simulink model "MX_Gateway"  (Simulink 9.0)
settings: Solver=FixedStepDiscrete  StartTime=0.0  StopTime=100  FixedStep=0.01  RelTol=1e-3
values from neighbouring files (symbols this model uses):
  ACTIVE = Bobcat.Parameter; CreateDefine( ACTIVE                         ,1                          ,'Define' ,'defines.h' )    [defines.m]
  ACTIVE_MODE_ONE = Bobcat.Parameter; CreateDefine( ACTIVE_MODE_ONE                ,19                         ,'Define' ,'defines.h' )    [defines.m]
  AUTHENTICATED = Bobcat.Parameter; CreateDefine( AUTHENTICATED                  ,1                          ,'Define' ,'defines.h' )    [defines.m]
  AUX_EMEA_CONTROLS = Bobcat.Parameter; CreateDefine( AUX_EMEA_CONTROLS  ,1   ,'Define' ,'defines.h' )    [enums.m]
  AUX_FLOW_HIGH = Bobcat.Parameter; CreateDefine( AUX_FLOW_HIGH           ,4  ,'Define' ,'defines.h' )    [enums.m]
  AUX_FLOW_INACTIVE = Bobcat.Parameter; CreateDefine( AUX_FLOW_INACTIVE       ,0  ,'Define' ,'defines.h' )    [enums.m]
  AUX_FLOW_LOW = Bobcat.Parameter; CreateDefine( AUX_FLOW_LOW            ,1  ,'Define' ,'defines.h' )    [enums.m]
  AUX_FLOW_MEDIUM = Bobcat.Parameter; CreateDefine( AUX_FLOW_MEDIUM         ,2  ,'Define' ,'defines.h' )    [enums.m]
  AUX_FLOW_STANDARD = Bobcat.Parameter; CreateDefine( AUX_FLOW_STANDARD       ,3  ,'Define' ,'defines.h' )    [enums.m]
  AUX_NON_FUNCTIONAL = Bobcat.Parameter; CreateDefine( AUX_NON_FUNCTIONAL ,255 ,'Define' ,'defines.h' )    [enums.m]
  BASE_ACTIVE = Bobcat.Parameter; CreateDefine( BASE_ACTIVE                    ,253                        ,'Define' ,'defines.h' )    [defines.m]
  BASE_DETENT = Bobcat.Parameter; CreateDefine( BASE_DETENT                    ,1                          ,'Define' ,'defines.h' )    [defines.m]
  BEEP_NONE = Bobcat.Parameter; CreateDefine( BEEP_NONE                      ,255                        ,'Define' ,'defines.h' )    [defines.m]
  CD_AIR_FILTER_DEBOUNCE_NORMAL = Bobcat.Parameter; CreateAliasCD( CD_AIR_FILTER_DEBOUNCE_NORMAL                        ,uint16(500)                ,'ConstantDataPointer' ,'CONSTANT_DATA' ,'AIR_FILTER_DEBOUNCE_NORMAL')    [cdata.m]    (also in hc12_cdata.m; m4_cdata.m)
  CD_AIR_FILTER_DEBOUNCE_NOT_CONNECTED = Bobcat.Parameter; CreateAliasCD( CD_AIR_FILTER_DEBOUNCE_NOT_CONNECTED                 ,uint16(3000)               ,'ConstantDataPointer' ,'CONSTANT_DATA' ,'AIR_FILTER_DEBOUNCE_NOT_CONNECTED')    [cdata.m]    (also in hc12_cdata.m; m4_cdata.m)
  CD_AIR_FILTER_DEBOUNCE_PLUGGED = Bobcat.Parameter; CreateAliasCD( CD_AIR_FILTER_DEBOUNCE_PLUGGED                       ,uint16(3000)               ,'ConstantDataPointer' ,'CONSTANT_DATA' ,'AIR_FILTER_DEBOUNCE_PLUGGED')    [cdata.m]    (also in hc12_cdata.m; m4_cdata.m)
  CD_AIR_FILTER_FUNCTION = Bobcat.Parameter; CreateAliasCD( CD_AIR_FILTER_FUNCTION                               ,uint8(255)                 ,'ConstantDataPointer' ,'CONSTANT_DATA' ,'AIR_FILTER_FUNCTION')    [cdata.m]    (also in hc12_cdata.m; m4_cdata.m)
  CD_AIR_FILTER_PLUGGED_WEIGHT = Bobcat.Parameter; CreateAliasCD( CD_AIR_FILTER_PLUGGED_WEIGHT                         ,uint8(2)                   ,'ConstantDataPointer' ,'CONSTANT_DATA' ,'AIR_FILTER_PLUGGED_WEIGHT')    [cdata.m]    (also in hc12_cdata.m; m4_cdata.m)
  CD_AIR_FILTER_UNPLUGGED_WEIGHT = Bobcat.Parameter; CreateAliasCD( CD_AIR_FILTER_UNPLUGGED_WEIGHT                       ,uint8(1)                   ,'ConstantDataPointer' ,'CONSTANT_DATA' ,'AIR_FILTER_UNPLUGGED_WEIGHT')    [cdata.m]    (also in hc12_cdata.m; m4_cdata.m)
  CD_AUTOIDLE_ARMBAR_DELAY = Bobcat.Parameter; CreateAliasCD( CD_AUTOIDLE_ARMBAR_DELAY                             ,uint8(2)                   ,'ConstantDataPointer' ,'CONSTANT_DATA' ,'AUTOIDLE_ARMBAR_DELAY')    [cdata.m]    (also in hc12_cdata.m; m4_cdata.m)
  CD_AUTOIDLE_FUNCTION = Bobcat.Parameter; CreateAliasCD( CD_AUTOIDLE_FUNCTION                                 ,uint8(255)                 ,'ConstantDataPointer' ,'CONSTANT_DATA' ,'AUTOIDLE_FUNCTION')    [cdata.m]    (also in hc12_cdata.m; m4_cdata.m)
  CD_AUTOIDLE_IDLE_DELAY = Bobcat.Parameter; CreateAliasCD( CD_AUTOIDLE_IDLE_DELAY                               ,uint8(40)                  ,'ConstantDataPointer' ,'CONSTANT_DATA' ,'AUTOIDLE_IDLE_DELAY')    [cdata.m]    (also in hc12_cdata.m; m4_cdata.m)
  CD_AUTOIDLE_LOW_IDLE_START = Bobcat.Parameter; CreateAliasCD( CD_AUTOIDLE_LOW_IDLE_START                           ,uint8(255)                 ,'ConstantDataPointer' ,'CONSTANT_DATA' ,'AUTOIDLE_LOW_IDLE_START')    [cdata.m]    (also in hc12_cdata.m; m4_cdata.m)
  CD_AUTOIDLE_PRESSURE_OFF = Bobcat.Parameter; CreateAliasCD( CD_AUTOIDLE_PRESSURE_OFF                             ,uint16(300)                ,'ConstantDataPointer' ,'CONSTANT_DATA' ,'AUTOIDLE_PRESSURE_OFF')    [cdata.m]    (also in hc12_cdata.m; m4_cdata.m)
  CD_AUTOIDLE_PRESSURE_ON = Bobcat.Parameter; CreateAliasCD( CD_AUTOIDLE_PRESSURE_ON                              ,uint16(270)                ,'ConstantDataPointer' ,'CONSTANT_DATA' ,'AUTOIDLE_PRESSURE_ON')    [cdata.m]    (also in hc12_cdata.m; m4_cdata.m)
  CD_AUTOSHIFT_FUNCTION = Bobcat.Parameter; CreateAliasCD( CD_AUTOSHIFT_FUNCTION                                ,uint8(255)                 ,'ConstantDataPointer' ,'CONSTANT_DATA' ,'AUTOSHIFT_FUNCTION')    [cdata.m]    (also in hc12_cdata.m; m4_cdata.m)
  CD_AUTOSHIFT_PHIGH1 = Bobcat.Parameter; CreateAliasCD( CD_AUTOSHIFT_PHIGH1                                  ,uint16(205)                ,'ConstantDataPointer' ,'CONSTANT_DATA' ,'AUTOSHIFT_PHIGH1')    [cdata.m]    (also in hc12_cdata.m; m4_cdata.m)
  CD_AUTOSHIFT_PHIGH2 = Bobcat.Parameter; CreateAliasCD( CD_AUTOSHIFT_PHIGH2                                  ,uint16(193)                ,'ConstantDataPointer' ,'CONSTANT_DATA' ,'AUTOSHIFT_PHIGH2')    [cdata.m]    (also in hc12_cdata.m; m4_cdata.m)
  CD_AUTOSHIFT_PHIGH_TEMP1 = Bobcat.Parameter; CreateAliasCD( CD_AUTOSHIFT_PHIGH_TEMP1                             ,int16(70)                  ,'ConstantDataPointer' ,'CONSTANT_DATA' ,'AUTOSHIFT_PHIGH_TEMP1')    [cdata.m]    (also in hc12_cdata.m; m4_cdata.m)
  CD_AUTOSHIFT_PHIGH_TEMP2 = Bobcat.Parameter; CreateAliasCD( CD_AUTOSHIFT_PHIGH_TEMP2                             ,int16(160)                 ,'ConstantDataPointer' ,'CONSTANT_DATA' ,'AUTOSHIFT_PHIGH_TEMP2')    [cdata.m]    (also in hc12_cdata.m; m4_cdata.m)
  CD_AUTOSHIFT_PLOW1 = Bobcat.Parameter; CreateAliasCD( CD_AUTOSHIFT_PLOW1                                   ,uint16(130)                ,'ConstantDataPointer' ,'CONSTANT_DATA' ,'AUTOSHIFT_PLOW1')    [cdata.m]    (also in hc12_cdata.m; m4_cdata.m)
  CD_AUTOSHIFT_PLOW2 = Bobcat.Parameter; CreateAliasCD( CD_AUTOSHIFT_PLOW2                                   ,uint16(100)                ,'ConstantDataPointer' ,'CONSTANT_DATA' ,'AUTOSHIFT_PLOW2')    [cdata.m]    (also in hc12_cdata.m; m4_cdata.m)
  CD_AUTOSHIFT_PLOW_TEMP1 = Bobcat.Parameter; CreateAliasCD( CD_AUTOSHIFT_PLOW_TEMP1                              ,int16(20)                  ,'ConstantDataPointer' ,'CONSTANT_DATA' ,'AUTOSHIFT_PLOW_TEMP1')    [cdata.m]    (also in hc12_cdata.m; m4_cdata.m)
  CD_AUTOSHIFT_PLOW_TEMP2 = Bobcat.Parameter; CreateAliasCD( CD_AUTOSHIFT_PLOW_TEMP2                              ,int16(80)                  ,'ConstantDataPointer' ,'CONSTANT_DATA' ,'AUTOSHIFT_PLOW_TEMP2')    [cdata.m]    (also in hc12_cdata.m; m4_cdata.m)
  CD_AUTOSHIFT_TRAVEL_DELAY = Bobcat.Parameter; CreateAliasCD( CD_AUTOSHIFT_TRAVEL_DELAY                            ,uint8(32)                  ,'ConstantDataPointer' ,'CONSTANT_DATA' ,'AUTOSHIFT_TRAVEL_DELAY')    [cdata.m]    (also in hc12_cdata.m; m4_cdata.m)
  CD_AUX2_CRACKPOINT_CURRENT = Bobcat.Parameter; CreateAliasCD( CD_AUX2_CRACKPOINT_CURRENT                           ,fi(0,0,16,12,'hex','0c28') ,'ConstantDataPointer' ,'CONSTANT_DATA' ,'AUX2_CRACKPOINT_CURRENT')    [cdata.m]    (also in hc12_cdata.m; m4_cdata.m)
  CD_AUX2_FLOW_LOW_FUNCTION = Bobcat.Parameter; CreateAliasCD( CD_AUX2_FLOW_LOW_FUNCTION                            ,uint8(255)                 ,'ConstantDataPointer' ,'CONSTANT_DATA' ,'AUX2_FLOW_LOW_FUNCTION')    [cdata.m]    (also in hc12_cdata.m; m4_cdata.m)
  CD_AUX2_FLOW_MED_FUNCTION = Bobcat.Parameter; CreateAliasCD( CD_AUX2_FLOW_MED_FUNCTION                            ,uint8(255)                 ,'ConstantDataPointer' ,'CONSTANT_DATA' ,'AUX2_FLOW_MED_FUNCTION')    [cdata.m]    (also in hc12_cdata.m; m4_cdata.m)
  CD_AUX2_HI_FLOW_PERCENT = Bobcat.Parameter; CreateAliasCD( CD_AUX2_HI_FLOW_PERCENT                              ,fi(0,0,16,16,'hex','ffff') ,'ConstantDataPointer' ,'CONSTANT_DATA' ,'AUX2_HI_FLOW_PERCENT')    [cdata.m]    (also in hc12_cdata.m; m4_cdata.m)
  CD_AUX2_LOW_FLOW_PERCENT = Bobcat.Parameter; CreateAliasCD( CD_AUX2_LOW_FLOW_PERCENT                             ,fi(0,0,16,16,'hex','7333') ,'ConstantDataPointer' ,'CONSTANT_DATA' ,'AUX2_LOW_FLOW_PERCENT')    [cdata.m]    (also in hc12_cdata.m; m4_cdata.m)
  CD_AUX2_MAX_FLOW_CURRENT = Bobcat.Parameter; CreateAliasCD( CD_AUX2_MAX_FLOW_CURRENT                             ,fi(0,0,16,12,'hex','14cc') ,'ConstantDataPointer' ,'CONSTANT_DATA' ,'AUX2_MAX_FLOW_CURRENT')    [cdata.m]    (also in hc12_cdata.m; m4_cdata.m)
  CD_AUX2_MED_FLOW_PERCENT = Bobcat.Parameter; CreateAliasCD( CD_AUX2_MED_FLOW_PERCENT                             ,fi(0,0,16,16,'hex','9999') ,'ConstantDataPointer' ,'CONSTANT_DATA' ,'AUX2_MED_FLOW_PERCENT')    [cdata.m]    (also in hc12_cdata.m; m4_cdata.m)
  CD_AUX_CRACKPOINT_CURRENT = Bobcat.Parameter; CreateAliasCD( CD_AUX_CRACKPOINT_CURRENT                            ,fi(0,0,16,12,'hex','0000') ,'ConstantDataPointer' ,'CONSTANT_DATA' ,'AUX_CRACKPOINT_CURRENT')    [cdata.m]    (also in hc12_cdata.m; m4_cdata.m)
  CD_AUX_DETENT_FLASH_TIME = Bobcat.Parameter; CreateAliasCD( CD_AUX_DETENT_FLASH_TIME                             ,uint16(72)                 ,'ConstantDataPointer' ,'CONSTANT_DATA' ,'AUX_DETENT_FLASH_TIME')    [cdata.m]    (also in hc12_cdata.m; m4_cdata.m)
  CD_AUX_DETENT_HOLD_TIME = Bobcat.Parameter; CreateAliasCD( CD_AUX_DETENT_HOLD_TIME                              ,uint16(100)                ,'ConstantDataPointer' ,'CONSTANT_DATA' ,'AUX_DETENT_HOLD_TIME')    [cdata.m]    (also in hc12_cdata.m; m4_cdata.m)
  CD_AUX_FULL_ON_AUX1 = Bobcat.Parameter; CreateAliasCD( CD_AUX_FULL_ON_AUX1                                  ,fi(0,0,16,12,'hex','10cd') ,'ConstantDataPointer' ,'CONSTANT_DATA' ,'AUX_FULL_ON_AUX1')    [cdata.m]    (also in hc12_cdata.m; m4_cdata.m)
  CD_AUX_FULL_ON_AUX2 = Bobcat.Parameter; CreateAliasCD( CD_AUX_FULL_ON_AUX2                                  ,fi(0,0,16,12,'hex','139d') ,'ConstantDataPointer' ,'CONSTANT_DATA' ,'AUX_FULL_ON_AUX2')    [cdata.m]    (also in hc12_cdata.m; m4_cdata.m)
  CD_AUX_FULL_ON_AUX3 = Bobcat.Parameter; CreateAliasCD( CD_AUX_FULL_ON_AUX3                                  ,fi(0,0,16,12,'hex','17d7') ,'ConstantDataPointer' ,'CONSTANT_DATA' ,'AUX_FULL_ON_AUX3')    [cdata.m]    (also in hc12_cdata.m; m4_cdata.m)
  CD_AUX_FUNCTION = Bobcat.Parameter; CreateAliasCD( CD_AUX_FUNCTION                                      ,uint8(0)                   ,'ConstantDataPointer' ,'CONSTANT_DATA' ,'AUX_FUNCTION')    [cdata.m]    (also in hc12_cdata.m; m4_cdata.m)
  CD_AUX_RATE_LIMIT_CRACKPOINT = Bobcat.Parameter; CreateAliasCD( CD_AUX_RATE_LIMIT_CRACKPOINT                         ,fi(0,0,16,12,'hex','0800') ,'ConstantDataPointer' ,'CONSTANT_DATA' ,'AUX_RATE_LIMIT_CRACKPOINT')    [cdata.m]    (also in hc12_cdata.m; m4_cdata.m)
  CD_AUX_RATE_LIMIT_CRACKPOINT_RATE = Bobcat.Parameter; CreateAliasCD( CD_AUX_RATE_LIMIT_CRACKPOINT_RATE                    ,fi(0,1,32,12,'int',102400) ,'ConstantDataPointer' ,'CONSTANT_DATA' ,'AUX_RATE_LIMIT_CRACKPOINT_RATE')    [cdata.m]    (also in hc12_cdata.m; m4_cdata.m)
  CD_AUX_RATE_LIMIT_FUNCTION = Bobcat.Parameter; CreateAliasCD( CD_AUX_RATE_LIMIT_FUNCTION                           ,uint8(255)                 ,'ConstantDataPointer' ,'CONSTANT_DATA' ,'AUX_RATE_LIMIT_FUNCTION')    [cdata.m]    (also in hc12_cdata.m; m4_cdata.m)
  CD_AUX_RATE_LIMIT_MAX_ENG_SPD = Bobcat.Parameter; CreateAliasCD( CD_AUX_RATE_LIMIT_MAX_ENG_SPD                        ,uint16(1600)               ,'ConstantDataPointer' ,'CONSTANT_DATA' ,'AUX_RATE_LIMIT_MAX_ENG_SPD')    [cdata.m]    (also in hc12_cdata.m; m4_cdata.m)
  CD_AUX_RATE_LIMIT_MIN_ENG_SPD = Bobcat.Parameter; CreateAliasCD( CD_AUX_RATE_LIMIT_MIN_ENG_SPD                        ,uint16(100)                ,'ConstantDataPointer' ,'CONSTANT_DATA' ,'AUX_RATE_LIMIT_MIN_ENG_SPD')    [cdata.m]    (also in hc12_cdata.m; m4_cdata.m)
  CD_AUX_RATE_LIMIT_NORMAL_RATE = Bobcat.Parameter; CreateAliasCD( CD_AUX_RATE_LIMIT_NORMAL_RATE                        ,fi(0,1,32,12,'int',2048)   ,'ConstantDataPointer' ,'CONSTANT_DATA' ,'AUX_RATE_LIMIT_NORMAL_RATE')    [cdata.m]    (also in hc12_cdata.m; m4_cdata.m)
  CD_AUXPOT_CAL_DEADBAND_HIGH = Bobcat.Parameter; CreateAliasCD( CD_AUXPOT_CAL_DEADBAND_HIGH                          ,uint16(574)                ,'ConstantDataPointer' ,'CONSTANT_DATA' ,'AUXPOT_CAL_DEADBAND_HIGH')    [cdata.m]    (also in hc12_cdata.m; m4_cdata.m)
  CD_AUXPOT_CAL_DEADBAND_LOW = Bobcat.Parameter; CreateAliasCD( CD_AUXPOT_CAL_DEADBAND_LOW                           ,uint16(424)                ,'ConstantDataPointer' ,'CONSTANT_DATA' ,'AUXPOT_CAL_DEADBAND_LOW')    [cdata.m]    (also in hc12_cdata.m; m4_cdata.m)
  CD_AUXPOT_CENTER_RATIO = Bobcat.Parameter; CreateAliasCD( CD_AUXPOT_CENTER_RATIO                               ,uint16(500)                ,'ConstantDataPointer' ,'CONSTANT_DATA' ,'AUXPOT_CENTER_RATIO')    [cdata.m]    (also in hc12_cdata.m; m4_cdata.m)
  CD_AUXPOT_DEADBAND = Bobcat.Parameter; CreateAliasCD( CD_AUXPOT_DEADBAND                                   ,uint8(38)                  ,'ConstantDataPointer' ,'CONSTANT_DATA' ,'AUXPOT_DEADBAND')    [cdata.m]    (also in hc12_cdata.m; m4_cdata.m)
  CD_AUXPOT_FUNCTION = Bobcat.Parameter; CreateAliasCD( CD_AUXPOT_FUNCTION                                   ,uint8(0)                   ,'ConstantDataPointer' ,'CONSTANT_DATA' ,'AUXPOT_FUNCTION')    [cdata.m]    (also in hc12_cdata.m; m4_cdata.m)
  CD_AUXPOT_RATIO_GAIN = Bobcat.Parameter; CreateAliasCD( CD_AUXPOT_RATIO_GAIN                                 ,uint16(1000)               ,'ConstantDataPointer' ,'CONSTANT_DATA' ,'AUXPOT_RATIO_GAIN')    [cdata.m]    (also in hc12_cdata.m; m4_cdata.m)
  CD_CAN_THROTTLE_FUNCTION = Bobcat.Parameter; CreateAliasCD( CD_CAN_THROTTLE_FUNCTION                             ,uint8(255)                 ,'ConstantDataPointer' ,'CONSTANT_DATA' ,'CAN_THROTTLE_FUNCTION')    [cdata.m]    (also in hc12_cdata.m; m4_cdata.m)
  CD_CAN_THROTTLE_REVERSE = Bobcat.Parameter; CreateAliasCD( CD_CAN_THROTTLE_REVERSE                              ,uint8(255)                 ,'ConstantDataPointer' ,'CONSTANT_DATA' ,'CAN_THROTTLE_REVERSE')    [cdata.m]    (also in hc12_cdata.m; m4_cdata.m)
  CD_CONSOLE_DEBOUNCE_ABSENT = Bobcat.Parameter; CreateAliasCD( CD_CONSOLE_DEBOUNCE_ABSENT                           ,uint16(12)                 ,'ConstantDataPointer' ,'CONSTANT_DATA' ,'CONSOLE_DEBOUNCE_ABSENT')    [cdata.m]    (also in hc12_cdata.m; m4_cdata.m)
  CD_CONSOLE_DEBOUNCE_OORH = Bobcat.Parameter; CreateAliasCD( CD_CONSOLE_DEBOUNCE_OORH                             ,uint16(12)                 ,'ConstantDataPointer' ,'CONSTANT_DATA' ,'CONSOLE_DEBOUNCE_OORH')    [cdata.m]    (also in hc12_cdata.m; m4_cdata.m)
  CD_CONSOLE_DEBOUNCE_OORL = Bobcat.Parameter; CreateAliasCD( CD_CONSOLE_DEBOUNCE_OORL                             ,uint16(12)                 ,'ConstantDataPointer' ,'CONSTANT_DATA' ,'CONSOLE_DEBOUNCE_OORL')    [cdata.m]    (also in hc12_cdata.m; m4_cdata.m)
  CD_CONSOLE_DEBOUNCE_PRESENT = Bobcat.Parameter; CreateAliasCD( CD_CONSOLE_DEBOUNCE_PRESENT                          ,uint16(12)                 ,'ConstantDataPointer' ,'CONSTANT_DATA' ,'CONSOLE_DEBOUNCE_PRESENT')    [cdata.m]    (also in hc12_cdata.m; m4_cdata.m)
  CD_CONSOLE_FUNCTION = Bobcat.Parameter; CreateAliasCD( CD_CONSOLE_FUNCTION                                  ,uint8(0)                   ,'ConstantDataPointer' ,'CONSTANT_DATA' ,'CONSOLE_FUNCTION')    [cdata.m]    (also in hc12_cdata.m; m4_cdata.m)
  CD_CONSOLE_OORH = Bobcat.Parameter; CreateAliasCD( CD_CONSOLE_OORH                                      ,uint16(740)                ,'ConstantDataPointer' ,'CONSTANT_DATA' ,'CONSOLE_OORH')    [cdata.m]    (also in hc12_cdata.m; m4_cdata.m)
  CD_CONSOLE_OORL = Bobcat.Parameter; CreateAliasCD( CD_CONSOLE_OORL                                      ,uint16(10)                 ,'ConstantDataPointer' ,'CONSTANT_DATA' ,'CONSOLE_OORL')    [cdata.m]    (also in hc12_cdata.m; m4_cdata.m)
  CD_CONSOLE_PRESENT_HIGH = Bobcat.Parameter; CreateAliasCD( CD_CONSOLE_PRESENT_HIGH                              ,uint16(515)                ,'ConstantDataPointer' ,'CONSTANT_DATA' ,'CONSOLE_PRESENT_HIGH')    [cdata.m]    (also in hc12_cdata.m; m4_cdata.m)
  CD_CONSOLE_PRESENT_LOW = Bobcat.Parameter; CreateAliasCD( CD_CONSOLE_PRESENT_LOW                               ,uint16(375)                ,'ConstantDataPointer' ,'CONSTANT_DATA' ,'CONSOLE_PRESENT_LOW')    [cdata.m]    (also in hc12_cdata.m; m4_cdata.m)
  CD_DELUXE_GEN5_5_FUNCTION = Bobcat.Parameter; CreateAliasCD( CD_DELUXE_GEN5_5_FUNCTION                            ,uint8(255)                 ,'ConstantDataPointer' ,'CONSTANT_DATA' ,'DELUXE_GEN5_5_FUNCTION')    [cdata.m]    (also in hc12_cdata.m; m4_cdata.m)
  CD_DELUXE_GEN5_FUNCTION = Bobcat.Parameter; CreateAliasCD( CD_DELUXE_GEN5_FUNCTION                              ,uint8(255)                 ,'ConstantDataPointer' ,'CONSTANT_DATA' ,'DELUXE_GEN5_FUNCTION')    [cdata.m]    (also in hc12_cdata.m; m4_cdata.m)
  CD_DERATE_ENGINE_SPEED_DEBOUNCE_HIGH = Bobcat.Parameter; CreateAliasCD( CD_DERATE_ENGINE_SPEED_DEBOUNCE_HIGH                 ,uint16(200)                ,'ConstantDataPointer' ,'CONSTANT_DATA' ,'DERATE_ENGINE_SPEED_DEBOUNCE_HIGH')    [cdata.m]    (also in hc12_cdata.m; m4_cdata.m)
  CD_DERATE_ENGINE_SPEED_DEBOUNCE_SHUTDOWN = Bobcat.Parameter; CreateAliasCD( CD_DERATE_ENGINE_SPEED_DEBOUNCE_SHUTDOWN             ,uint16(1000)               ,'ConstantDataPointer' ,'CONSTANT_DATA' ,'DERATE_ENGINE_SPEED_DEBOUNCE_SHUTDOWN')    [cdata.m]    (also in hc12_cdata.m; m4_cdata.m)
  CD_DERATE_ENGINE_SPEED_HIGH_OFFSET = Bobcat.Parameter; CreateAliasCD( CD_DERATE_ENGINE_SPEED_HIGH_OFFSET                   ,uint16(200)                ,'ConstantDataPointer' ,'CONSTANT_DATA' ,'DERATE_ENGINE_SPEED_HIGH_OFFSET')    [cdata.m]    (also in hc12_cdata.m; m4_cdata.m)
  CD_DERATE_METHOD = Bobcat.Parameter; CreateAliasCD( CD_DERATE_METHOD                                     ,uint8(0)                   ,'ConstantDataPointer' ,'CONSTANT_DATA' ,'DERATE_METHOD')    [cdata.m]    (also in hc12_cdata.m; m4_cdata.m)
  CD_DUMP_VALVE_FUNCTION = Bobcat.Parameter; CreateAliasCD( CD_DUMP_VALVE_FUNCTION                               ,uint8(255)                 ,'ConstantDataPointer' ,'CONSTANT_DATA' ,'DUMP_VALVE_FUNCTION')    [cdata.m]    (also in hc12_cdata.m; m4_cdata.m)
  CD_DUMP_VALVE_ON_CURRENT = Bobcat.Parameter; CreateAliasCD( CD_DUMP_VALVE_ON_CURRENT                             ,fi(0,0,16,12,'hex','ffff') ,'ConstantDataPointer' ,'CONSTANT_DATA' ,'DUMP_VALVE_ON_CURRENT')    [cdata.m]    (also in hc12_cdata.m; m4_cdata.m)
  CD_ECO_MODE_THROTTLE_REDUCTION = Bobcat.Parameter; CreateAliasCD( CD_ECO_MODE_THROTTLE_REDUCTION                       ,uint16(850)                ,'ConstantDataPointer' ,'CONSTANT_DATA' ,'ECO_MODE_THROTTLE_REDUCTION')    [cdata.m]    (also in hc12_cdata.m; m4_cdata.m)
  CD_ECT_FILTER_GAIN = Bobcat.Parameter; CreateAliasCD( CD_ECT_FILTER_GAIN                                   ,uint8(6)                   ,'ConstantDataPointer' ,'CONSTANT_DATA' ,'ECT_FILTER_GAIN')    [cdata.m]    (also in hc12_cdata.m; m4_cdata.m)
  CD_ECU_FUNCTION = Bobcat.Parameter; CreateAliasCD( CD_ECU_FUNCTION                                      ,uint8(255)                 ,'ConstantDataPointer' ,'CONSTANT_DATA' ,'ECU_FUNCTION')    [cdata.m]    (also in hc12_cdata.m; m4_cdata.m)
  CD_ECU_HOLD_DEBOUNCE_ERROR_ON = Bobcat.Parameter; CreateAliasCD( CD_ECU_HOLD_DEBOUNCE_ERROR_ON                        ,uint16(20)                 ,'ConstantDataPointer' ,'CONSTANT_DATA' ,'ECU_HOLD_DEBOUNCE_ERROR_ON')    [cdata.m]    (also in hc12_cdata.m; m4_cdata.m)
  CD_EMEA_CONTROLS_HOLD_TIME = Bobcat.Parameter; CreateAliasCD( CD_EMEA_CONTROLS_HOLD_TIME                           ,uint16(30)                 ,'ConstantDataPointer' ,'CONSTANT_DATA' ,'EMEA_CONTROLS_HOLD_TIME')    [cdata.m]    (also in hc12_cdata.m; m4_cdata.m)
  CD_EMEA_CONTROLS_OPTION_A = Bobcat.Parameter; CreateAliasCD( CD_EMEA_CONTROLS_OPTION_A                            ,uint8(255)                 ,'ConstantDataPointer' ,'CONSTANT_DATA' ,'EMEA_CONTROLS_OPTION_A')    [cdata.m]    (also in hc12_cdata.m; m4_cdata.m)
  CD_EMEA_CONTROLS_OPTION_C = Bobcat.Parameter; CreateAliasCD( CD_EMEA_CONTROLS_OPTION_C                            ,uint8(255)                 ,'ConstantDataPointer' ,'CONSTANT_DATA' ,'EMEA_CONTROLS_OPTION_C')    [cdata.m]    (also in hc12_cdata.m; m4_cdata.m)
  CD_EMEA_CONTROLS_REVERSE = Bobcat.Parameter; CreateAliasCD( CD_EMEA_CONTROLS_REVERSE                             ,uint8(255)                 ,'ConstantDataPointer' ,'CONSTANT_DATA' ,'EMEA_CONTROLS_REVERSE')    [cdata.m]    (also in hc12_cdata.m; m4_cdata.m)
  CD_EMEA_ELECTRIC_VERSION = Bobcat.Parameter; CreateAliasCD( CD_EMEA_ELECTRIC_VERSION                             ,uint8(0)                   ,'ConstantDataPointer' ,'CONSTANT_DATA' ,'EMEA_ELECTRIC_VERSION')    [cdata.m]    (also in hc12_cdata.m; m4_cdata.m)
  CD_EMEA_POT_CAL_DEADBAND_HIGH = Bobcat.Parameter; CreateAliasCD( CD_EMEA_POT_CAL_DEADBAND_HIGH                        ,uint16(574)                ,'ConstantDataPointer' ,'CONSTANT_DATA' ,'EMEA_POT_CAL_DEADBAND_HIGH')    [cdata.m]    (also in hc12_cdata.m; m4_cdata.m)
  CD_EMEA_POT_CAL_DEADBAND_LOW = Bobcat.Parameter; CreateAliasCD( CD_EMEA_POT_CAL_DEADBAND_LOW                         ,uint16(424)                ,'ConstantDataPointer' ,'CONSTANT_DATA' ,'EMEA_POT_CAL_DEADBAND_LOW')    [cdata.m]    (also in hc12_cdata.m; m4_cdata.m)
  CD_ENGINE_RPM_FACTOR = Bobcat.Parameter; CreateAliasCD( CD_ENGINE_RPM_FACTOR                                 ,uint8(35)                  ,'ConstantDataPointer' ,'CONSTANT_DATA' ,'ENGINE_RPM_FACTOR')    [cdata.m]    (also in hc12_cdata.m; m4_cdata.m)
  CD_ENGINE_SPEED_FILTER_FAST_VALUE = Bobcat.Parameter; CreateAliasCD( CD_ENGINE_SPEED_FILTER_FAST_VALUE                    ,uint8(1)                   ,'ConstantDataPointer' ,'CONSTANT_DATA' ,'ENGINE_SPEED_FILTER_FAST_VALUE')    [cdata.m]    (also in hc12_cdata.m; m4_cdata.m)
  CD_ENGINE_SPEED_FILTER_SLOW_VALUE = Bobcat.Parameter; CreateAliasCD( CD_ENGINE_SPEED_FILTER_SLOW_VALUE                    ,uint8(4)                   ,'ConstantDataPointer' ,'CONSTANT_DATA' ,'ENGINE_SPEED_FILTER_SLOW_VALUE')    [cdata.m]    (also in hc12_cdata.m; m4_cdata.m)
  CD_ENGINE_SPEED_RUNNING = Bobcat.Parameter; CreateAliasCD( CD_ENGINE_SPEED_RUNNING                              ,uint16(800)                ,'ConstantDataPointer' ,'CONSTANT_DATA' ,'ENGINE_SPEED_RUNNING')    [cdata.m]    (also in hc12_cdata.m; m4_cdata.m)
  CD_ENGINE_START_DELAY_DEBOUNCE_RPM_NO_SIGNAL = Bobcat.Parameter; CreateAliasCD( CD_ENGINE_START_DELAY_DEBOUNCE_RPM_NO_SIGNAL         ,uint16(0)                  ,'ConstantDataPointer' ,'CONSTANT_DATA' ,'ENGINE_START_DELAY_DEBOUNCE_RPM_NO_SIGNAL')    [cdata.m]    (also in hc12_cdata.m; m4_cdata.m)
  CD_ENGINE_START_DELAY_OFF_TIME = Bobcat.Parameter; CreateAliasCD( CD_ENGINE_START_DELAY_OFF_TIME                       ,uint16(0)                  ,'ConstantDataPointer' ,'CONSTANT_DATA' ,'ENGINE_START_DELAY_OFF_TIME')    [cdata.m]    (also in hc12_cdata.m; m4_cdata.m)
  CD_ENGINE_START_DELAY_RPM_NO_SIGNAL = Bobcat.Parameter; CreateAliasCD( CD_ENGINE_START_DELAY_RPM_NO_SIGNAL                  ,uint16(0)                  ,'ConstantDataPointer' ,'CONSTANT_DATA' ,'ENGINE_START_DELAY_RPM_NO_SIGNAL')    [cdata.m]    (also in hc12_cdata.m; m4_cdata.m)
  CD_EOP_DEBOUNCE_EXTREMELY_LOW = Bobcat.Parameter; CreateAliasCD( CD_EOP_DEBOUNCE_EXTREMELY_LOW                        ,uint16(50)                 ,'ConstantDataPointer' ,'CONSTANT_DATA' ,'EOP_DEBOUNCE_EXTREMELY_LOW')    [cdata.m]    (also in hc12_cdata.m; m4_cdata.m)
  CD_EOP_DEBOUNCE_NORMAL = Bobcat.Parameter; CreateAliasCD( CD_EOP_DEBOUNCE_NORMAL                               ,uint16(50)                 ,'ConstantDataPointer' ,'CONSTANT_DATA' ,'EOP_DEBOUNCE_NORMAL')    [cdata.m]    (also in hc12_cdata.m; m4_cdata.m)
  CD_EOP_DEBOUNCE_SHUTDOWN = Bobcat.Parameter; CreateAliasCD( CD_EOP_DEBOUNCE_SHUTDOWN                             ,uint16(100)                ,'ConstantDataPointer' ,'CONSTANT_DATA' ,'EOP_DEBOUNCE_SHUTDOWN')    [cdata.m]    (also in hc12_cdata.m; m4_cdata.m)
  CD_EOP_FILTER_VALUE = Bobcat.Parameter; CreateAliasCD( CD_EOP_FILTER_VALUE                                  ,uint8(3)                   ,'ConstantDataPointer' ,'CONSTANT_DATA' ,'EOP_FILTER_VALUE')    [cdata.m]    (also in hc12_cdata.m; m4_cdata.m)
  CD_EOP_FUNCTION = Bobcat.Parameter; CreateAliasCD( CD_EOP_FUNCTION                                      ,uint8(1)                   ,'ConstantDataPointer' ,'CONSTANT_DATA' ,'EOP_FUNCTION')    [cdata.m]    (also in hc12_cdata.m; m4_cdata.m)
  CD_EOP_SWITCH_GAUGE_VALUE = Bobcat.Parameter; CreateAliasCD( CD_EOP_SWITCH_GAUGE_VALUE                            ,uint16(125)                ,'ConstantDataPointer' ,'CONSTANT_DATA' ,'EOP_SWITCH_GAUGE_VALUE')    [cdata.m]    (also in hc12_cdata.m; m4_cdata.m)
  CD_EOP_SWITCH_LOW = Bobcat.Parameter; CreateAliasCD( CD_EOP_SWITCH_LOW                                    ,uint16(50)                 ,'ConstantDataPointer' ,'CONSTANT_DATA' ,'EOP_SWITCH_LOW')    [cdata.m]    (also in hc12_cdata.m; m4_cdata.m)
  CD_EOP_SWITCH_PRESSURE_VALUE = Bobcat.Parameter; CreateAliasCD( CD_EOP_SWITCH_PRESSURE_VALUE                         ,uint16(40)                 ,'ConstantDataPointer' ,'CONSTANT_DATA' ,'EOP_SWITCH_PRESSURE_VALUE')    [cdata.m]    (also in hc12_cdata.m; m4_cdata.m)
  CD_EOT_DEBOUNCE_EXTREMELY_LOW = Bobcat.Parameter; CreateAliasCD( CD_EOT_DEBOUNCE_EXTREMELY_LOW                        ,uint16(2)                  ,'ConstantDataPointer' ,'CONSTANT_DATA' ,'EOT_DEBOUNCE_EXTREMELY_LOW')    [cdata.m]    (also in hc12_cdata.m; m4_cdata.m)
  CD_EOT_DEBOUNCE_LOW = Bobcat.Parameter; CreateAliasCD( CD_EOT_DEBOUNCE_LOW                                  ,uint16(2)                  ,'ConstantDataPointer' ,'CONSTANT_DATA' ,'EOT_DEBOUNCE_LOW')    [cdata.m]    (also in hc12_cdata.m; m4_cdata.m)
  CD_EOT_DEBOUNCE_NORMAL = Bobcat.Parameter; CreateAliasCD( CD_EOT_DEBOUNCE_NORMAL                               ,uint16(2)                  ,'ConstantDataPointer' ,'CONSTANT_DATA' ,'EOT_DEBOUNCE_NORMAL')    [cdata.m]    (also in hc12_cdata.m; m4_cdata.m)
  CD_EOT_EXTREMELY_LOW = Bobcat.Parameter; CreateAliasCD( CD_EOT_EXTREMELY_LOW                                 ,uint16(81)                 ,'ConstantDataPointer' ,'CONSTANT_DATA' ,'EOT_EXTREMELY_LOW')    [cdata.m]    (also in hc12_cdata.m; m4_cdata.m)
  CD_EOT_FUNCTION = Bobcat.Parameter; CreateAliasCD( CD_EOT_FUNCTION                                      ,uint8(255)                 ,'ConstantDataPointer' ,'CONSTANT_DATA' ,'EOT_FUNCTION')    [cdata.m]    (also in hc12_cdata.m; m4_cdata.m)
  CD_EOT_LOW = Bobcat.Parameter; CreateAliasCD( CD_EOT_LOW                                           ,uint16(90)                 ,'ConstantDataPointer' ,'CONSTANT_DATA' ,'EOT_LOW')    [cdata.m]    (also in hc12_cdata.m; m4_cdata.m)
  CD_EOT_TEMPERATURE_HYSTERESIS = Bobcat.Parameter; CreateAliasCD( CD_EOT_TEMPERATURE_HYSTERESIS                        ,uint16(2)                  ,'ConstantDataPointer' ,'CONSTANT_DATA' ,'EOT_TEMPERATURE_HYSTERESIS')    [cdata.m]    (also in hc12_cdata.m; m4_cdata.m)
  CD_FAST_TRAVEL_FUNCTION = Bobcat.Parameter; CreateAliasCD( CD_FAST_TRAVEL_FUNCTION                              ,uint8(255)                 ,'ConstantDataPointer' ,'CONSTANT_DATA' ,'FAST_TRAVEL_FUNCTION')    [cdata.m]    (also in hc12_cdata.m; m4_cdata.m)
  CD_FAST_TRAVEL_THROTTLE_DELAY = Bobcat.Parameter; CreateAliasCD( CD_FAST_TRAVEL_THROTTLE_DELAY                        ,uint16(10)                 ,'ConstantDataPointer' ,'CONSTANT_DATA' ,'FAST_TRAVEL_THROTTLE_DELAY')    [cdata.m]    (also in hc12_cdata.m; m4_cdata.m)
  CD_FAST_TRAVEL_THROTTLE_LIMIT = Bobcat.Parameter; CreateAliasCD( CD_FAST_TRAVEL_THROTTLE_LIMIT                        ,uint16(1000)               ,'ConstantDataPointer' ,'CONSTANT_DATA' ,'FAST_TRAVEL_THROTTLE_LIMIT')    [cdata.m]    (also in hc12_cdata.m; m4_cdata.m)
  CD_FLYWHEEL_TEETH = Bobcat.Parameter; CreateAliasCD( CD_FLYWHEEL_TEETH                                    ,uint8(83)                  ,'ConstantDataPointer' ,'CONSTANT_DATA' ,'FLYWHEEL_TEETH')    [cdata.m]    (also in hc12_cdata.m; m4_cdata.m)
  CD_FUEL_LEVEL_FILTER_FAST_VALUE = Bobcat.Parameter; CreateAliasCD( CD_FUEL_LEVEL_FILTER_FAST_VALUE                      ,uint8(5)                   ,'ConstantDataPointer' ,'CONSTANT_DATA' ,'FUEL_LEVEL_FILTER_FAST_VALUE')    [cdata.m]    (also in hc12_cdata.m; m4_cdata.m)
  CD_FUEL_LEVEL_FILTER_SLOW_VALUE = Bobcat.Parameter; CreateAliasCD( CD_FUEL_LEVEL_FILTER_SLOW_VALUE                      ,uint8(10)                  ,'ConstantDataPointer' ,'CONSTANT_DATA' ,'FUEL_LEVEL_FILTER_SLOW_VALUE')    [cdata.m]    (also in hc12_cdata.m; m4_cdata.m)
  CD_FUEL_PRIMARY_FEEDBACK_FUNCTION = Bobcat.Parameter; CreateAliasCD( CD_FUEL_PRIMARY_FEEDBACK_FUNCTION                    ,uint8(0)                   ,'ConstantDataPointer' ,'CONSTANT_DATA' ,'FUEL_PRIMARY_FEEDBACK_FUNCTION')    [cdata.m]    (also in hc12_cdata.m; m4_cdata.m)
  ... (+246 more)
undefined symbols (not defined in the file or in its folder): sigbuilder_block
undefined symbols (not defined in the file): CD_FUEL_RESOLUTION_TIMER
libraries: simulink x186, MX_Library x107
(part 22 of 48)

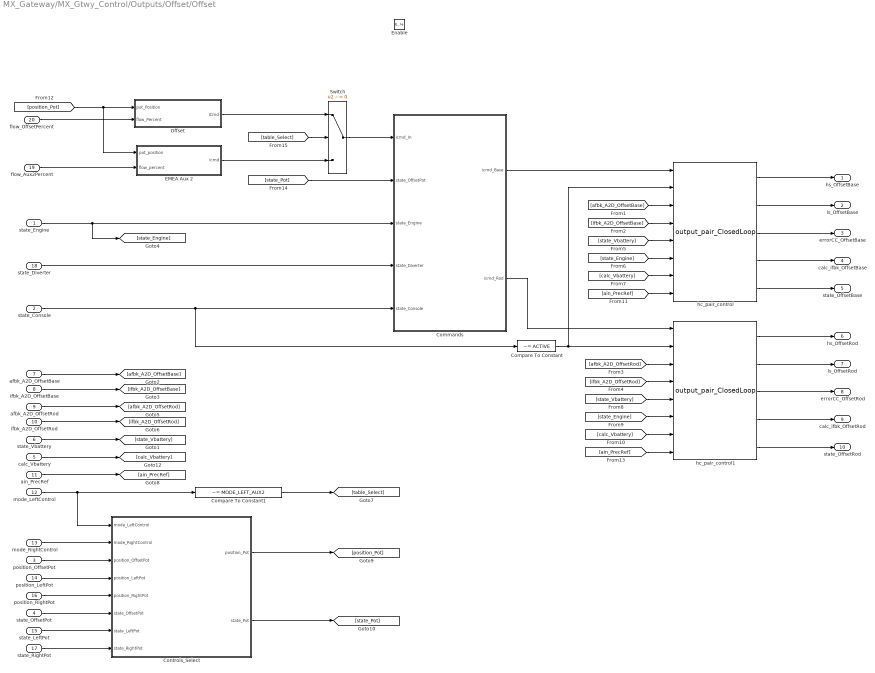
== system MX_Gateway/MX_Gtwy_Control/Outputs/Offset/Offset ==
  state_Engine : Inport
  state_Console : Inport  Port=2
  position_OffsetPot : Inport  Port=3
  state_OffsetPot : Inport  Port=4
  calc_Vbattery : Inport  Port=5
  state_Vbattery : Inport  Port=6
  afbk_A2D_OffsetBase : Inport  Port=7
  ifbk_A2D_OffsetBase : Inport  Port=8
  afbk_A2D_OffsetRod : Inport  Port=9
  ifbk_A2D_OffsetRod : Inport  Port=10
  ain_PrecRef : Inport  Port=11
  mode_LeftControl : Inport  Port=12
  mode_RightControl : Inport  Port=13
  position_LeftPot : Inport  Port=14
  state_LeftPot : Inport  Port=15
  position_RightPot : Inport  Port=16
  state_RightPot : Inport  Port=17
  state_Diverter : Inport  Port=18
  flow_Aux2Percent : Inport  Port=19
  flow_OffsetPercent : Inport  Port=20
  Enable : EnablePort  StatesWhenEnabling=reset  PropagateVarSize=During execution
  Commands : Stateflow chart  TreatAsAtomicUnit=on
  Compare To Constant : simulink/Logic and Bit Operations/Compare To Constant  relop=~=  const=ACTIVE  OutDataTypeStr=boolean
  Compare To Constant1 : simulink/Logic and Bit Operations/Compare To Constant  relop=~=  const=MODE_LEFT_AUX2  OutDataTypeStr=boolean
  Controls_Select : Truth Table  TreatAsAtomicUnit=on
  EMEA Aux 2 : Subsystem
  Offset : Subsystem
  Switch : Switch  Criteria=u2 ~= 0
  hc_pair_control : MX_Library/output_pair_ClosedLoop  AFBK_GAIN=CD_OFFSET_AFBK_GAIN  IFBK_GAIN=CD_OFFSET_IFBK_GAIN  OPEN_DB_CNT=CD_OFFSET_DEBOUNCE_OPEN_CIRCUIT  STG_DB_CNT=CD_OFFSET_DEBOUNCE_SHORT_TO_GROUND  OSTG_DB_CNT=CD_OFFSET_DEBOUNCE_SHORT_TO_GROUND  STB_DB_CNT=CD_OFFSET_DEBOUNCE_SHORT_TO_BATTERY  OC_DB_CNT=CD_OFFSET_DEBOUNCE_OVERCURRENT  IFBK_MAX=CD_OFFSET_OVERCURRENT  NZ_IFBK=CD_OFFSET_NON_ZERO_CURRENT  NZ_DUTY=CD_OFFSET_NON_ZERO_DUTY  KP=CD_OFFSET_KP  KI=CD_OFFSET_KI  BIDIRECTIONAL_CTRL=CD_OFFSET_BIDIRECTIONAL_CONTROL
  hc_pair_control1 : MX_Library/output_pair_ClosedLoop  AFBK_GAIN=CD_OFFSET_AFBK_GAIN  IFBK_GAIN=CD_OFFSET_IFBK_GAIN  OPEN_DB_CNT=CD_OFFSET_DEBOUNCE_OPEN_CIRCUIT  STG_DB_CNT=CD_OFFSET_DEBOUNCE_SHORT_TO_GROUND  OSTG_DB_CNT=CD_OFFSET_DEBOUNCE_SHORT_TO_GROUND  STB_DB_CNT=CD_OFFSET_DEBOUNCE_SHORT_TO_BATTERY  OC_DB_CNT=CD_OFFSET_DEBOUNCE_OVERCURRENT  IFBK_MAX=CD_OFFSET_OVERCURRENT  NZ_IFBK=CD_OFFSET_NON_ZERO_CURRENT  NZ_DUTY=CD_OFFSET_NON_ZERO_DUTY  KP=CD_OFFSET_KP  KI=CD_OFFSET_KI  BIDIRECTIONAL_CTRL=CD_OFFSET_BIDIRECTIONAL_CONTROL
  hs_OffsetBase : Outport  OutputWhenDisabled=reset  InitialOutput=[0]
  ls_OffsetBase : Outport  Port=2  OutputWhenDisabled=reset  InitialOutput=[0]
  errorCC_OffsetBase : Outport  Port=3  OutputWhenDisabled=reset  InitialOutput=[0]
  calc_ifbk_OffsetBase : Outport  Port=4  OutputWhenDisabled=reset  InitialOutput=[0]
  state_OffsetBase : Outport  Port=5  OutputWhenDisabled=reset  InitialOutput=[0]
  hs_OffsetRod : Outport  Port=6  OutputWhenDisabled=reset  InitialOutput=[0]
  ls_OffsetRod : Outport  Port=7  OutputWhenDisabled=reset  InitialOutput=[0]
  errorCC_OffsetRod : Outport  Port=8  OutputWhenDisabled=reset  InitialOutput=[0]
  calc_ifbk_OffsetRod : Outport  Port=9  OutputWhenDisabled=reset  InitialOutput=[0]
  state_OffsetRod : Outport  Port=10  InitialOutput=[0]
  wires:
  state_Engine/1 -> Commands/3(state_Engine), [state_Engine]
  state_Console/1 -> Commands/5(state_Console), Compare To Constant/1
  afbk_A2D_OffsetBase/1 -> [afbk_A2D_OffsetBase]
  ifbk_A2D_OffsetBase/1 -> [ifbk_A2D_OffsetBase]
  state_Vbattery/1 -> [state_Vbattery]
  afbk_A2D_OffsetRod/1 -> [afbk_A2D_OffsetRod]
  ifbk_A2D_OffsetRod/1 -> [ifbk_A2D_OffsetRod]
  calc_Vbattery/1 -> [calc_Vbattery]
  Commands/1(icmd_Base) -> hc_pair_control/1
  hc_pair_control/1 -> hs_OffsetBase/1
  hc_pair_control/2 -> ls_OffsetBase/1
  hc_pair_control/3 -> errorCC_OffsetBase/1
  hc_pair_control/4 -> calc_ifbk_OffsetBase/1
  hc_pair_control/5 -> state_OffsetBase/1
  hc_pair_control1/1 -> hs_OffsetRod/1
  hc_pair_control1/2 -> ls_OffsetRod/1
  hc_pair_control1/3 -> errorCC_OffsetRod/1
  hc_pair_control1/4 -> calc_ifbk_OffsetRod/1
  hc_pair_control1/5 -> state_OffsetRod/1
  Commands/2(icmd_Rod) -> hc_pair_control1/1
  [afbk_A2D_OffsetBase] -> hc_pair_control/3
  [ifbk_A2D_OffsetBase] -> hc_pair_control/4
  [state_Vbattery] -> hc_pair_control/5
  [state_Engine] -> hc_pair_control/6
  [calc_Vbattery] -> hc_pair_control/7
  [afbk_A2D_OffsetRod] -> hc_pair_control1/3
  [ifbk_A2D_OffsetRod] -> hc_pair_control1/4
  [state_Vbattery] -> hc_pair_control1/5
  [state_Engine] -> hc_pair_control1/6
  [calc_Vbattery] -> hc_pair_control1/7
  Compare To Constant/1 -> hc_pair_control1/2, hc_pair_control/2
  ain_PrecRef/1 -> [ain_PrecRef]
  [ain_PrecRef] -> hc_pair_control1/8
  [ain_PrecRef] -> hc_pair_control/8
  [position_Pot] -> EMEA Aux 2/1(pot_position), Offset/1(pot_Position)
  Switch/1 -> Commands/1(icmd_In)
  EMEA Aux 2/1(icmd) -> Switch/3
  mode_LeftControl/1 -> Controls_Select/1(mode_LeftControl), Compare To Constant1/1
  Controls_Select/1(position_Pot) -> [position_Pot]
  Controls_Select/2(state_Pot) -> [state_Pot]
  mode_RightControl/1 -> Controls_Select/2(mode_RightControl)
  Compare To Constant1/1 -> [table_Select]
  position_OffsetPot/1 -> Controls_Select/3(position_OffsetPot)
  position_RightPot/1 -> Controls_Select/5(position_RightPot)
  position_LeftPot/1 -> Controls_Select/4(position_LeftPot)
  state_OffsetPot/1 -> Controls_Select/6(state_OffsetPot)
  state_RightPot/1 -> Controls_Select/8(state_RightPot)
  state_LeftPot/1 -> Controls_Select/7(state_LeftPot)
  [table_Select] -> Switch/2
  [state_Pot] -> Commands/2(state_OffsetPot)
  state_Diverter/1 -> Commands/4(state_Diverter)
  Offset/1(iCmd) -> Switch/1
  flow_Aux2Percent/1 -> EMEA Aux 2/2(flow_percent)
  flow_OffsetPercent/1 -> Offset/2(flow_Percent)

== system MX_Gateway/MX_Gtwy_Control/Outputs/Two_Speed/Two_Speed ==
  state_Engine : Inport
  state_ECT : Inport  Port=2
  state_HOT : Inport  Port=3
  state_HydEnable : Inport  Port=4
  state_Console : Inport  Port=5
  state_LoadSense : Inport  Port=6
  flt_HOT : Inport  Port=7
  din_TravelPressureSwitch : Inport  Port=8
  afbk_TwoSpeed : Inport  Port=9
  state_Vbattery : Inport  Port=10
  calc_Vbattery : Inport  Port=11
  bar_LoadSense : Inport  Port=12
  din_TwoSpeedSwitch : Inport  Port=13
  Enable : EnablePort  StatesWhenEnabling=reset  PropagateVarSize=During execution
  DetectRisingEdge : simulink/Logic and Bit Operations/Detect Increase  vinit=0.0  InputProcessing=Inherited  OutDataTypeStr=uint8
  Pressure Calc : Subsystem
  TwoSpeed_Control : Stateflow chart  TreatAsAtomicUnit=on
  hc_Output : MX_Library/output_single_OpenLoop  FUNCTION=CD_TWOSPEED_FUNCTION  AFBK_GAIN=CD_TWOSPEED_AFBK_GAIN  OPEN_DB_CNT=CD_TWOSPEED_DEBOUNCE_OPEN_CIRCUIT  STB_DB_CNT=CD_TWOSPEED_DEBOUNCE_SHORT_TO_BATTERY  STG_DB_CNT=CD_TWOSPEED_DEBOUNCE_SHORT_TO_GROUND  MAX_VOLTS=CD_TWOSPEED_MAX_VOLTS
  state_TwoSpeed : Outport  OutputWhenDisabled=reset  InitialOutput=[0]
  state_TwoSpeedIcon : Outport  Port=2  OutputWhenDisabled=reset  InitialOutput=[0]
  duty_TwoSpeed : Outport  Port=3  OutputWhenDisabled=reset  InitialOutput=[0]
  wires:
  din_TwoSpeedSwitch/1 -> DetectRisingEdge/1
  state_ECT/1 -> TwoSpeed_Control/2(state_ECT)
  state_HOT/1 -> TwoSpeed_Control/9(state_HOT), Pressure Calc/1(state_HOT)
  state_Console/1 -> TwoSpeed_Control/3(state_Console)
  state_Engine/1 -> TwoSpeed_Control/4(state_Engine)
  state_HydEnable/1 -> TwoSpeed_Control/5(state_HydEnable)
  DetectRisingEdge/1 -> TwoSpeed_Control/1(risingedge_TwoSpeed)
  din_TravelPressureSwitch/1 -> TwoSpeed_Control/7(din_TravelPressureSwitch)
  bar_LoadSense/1 -> TwoSpeed_Control/6(pressure_LoadSense)
  state_LoadSense/1 -> TwoSpeed_Control/8(state_LoadSense)
  Pressure Calc/1(P_high) -> TwoSpeed_Control/10(P_high)
  Pressure Calc/2(P_low) -> TwoSpeed_Control/11(P_low)
  flt_HOT/1 -> Pressure Calc/2(flt_HOT)
  TwoSpeed_Control/1(state_TwoSpeedIcon) -> state_TwoSpeedIcon/1
  hc_Output/1 -> state_TwoSpeed/1
  hc_Output/2 -> duty_TwoSpeed/1
  TwoSpeed_Control/2(duty_TwoSpeed) -> hc_Output/1
  afbk_TwoSpeed/1 -> hc_Output/4
  state_Vbattery/1 -> hc_Output/2
  calc_Vbattery/1 -> hc_Output/3

== system MX_Gateway/MX_Gtwy_Control/System/AutoIdle/Auto_Idle_Enable ==
  button_AutoIdle : Inport
  state_LoadSense : Inport  Port=2
  flag_InitEERead : Inport  Port=3
  dlx_G55_AutoIdleEnable : Inport  Port=4
  Auto_Idle : Stateflow chart  TreatAsAtomicUnit=on
  DetectIncrease : simulink/Logic and Bit Operations/Detect Increase  vinit=1  InputProcessing=Inherited  OutDataTypeStr=uint8
  state_AutoIdleEnable : Outport  OutputWhenDisabled=reset
  wires:
  DetectIncrease/1 -> Auto_Idle/1(AutoIdle_RisingEdge)
  Auto_Idle/1(state_AutoIdleEnable) -> state_AutoIdleEnable/1
  state_LoadSense/1 -> Auto_Idle/2(state_LoadSense)
  button_AutoIdle/1 -> DetectIncrease/1
  flag_InitEERead/1 -> Auto_Idle/3(flag_InitEERead)
  dlx_G55_AutoIdleEnable/1 -> Auto_Idle/4(dlx_G55_AutoIdleEnable)

== system MX_Gateway/MX_Gtwy_Control/System/AutoIdle/Auto_Idle_Function ==
  psi_LoadSense : Inport
  state_Console : Inport  Port=2
  state_AutoIdleEnable : Inport  Port=3
  state_Engine : Inport  Port=4
  percent_Throttle : Inport  Port=5
  AutoIdleTime : Inport  Port=6
  Enable : EnablePort  StatesWhenEnabling=reset
  Auto_Idle : Stateflow chart  TreatAsAtomicUnit=on
  CompareToConstant1 : simulink/Logic and Bit Operations/Compare To Constant  relop===  const=NON_FUNCTIONAL  OutDataTypeStr=boolean  ZeroCross=off
  Constant1 : Constant  Value=CD_THROTTLE_PRIMARY_FUNCTION  OutDataTypeStr=uint8
  Constant2 : Constant  Value=WORD_UNAVAIL  OutDataTypeStr=uint16
  Switch : Switch  Criteria=u2 ~= 0
  cmd_AutoIdle : Outport  InitialOutput=[0]
  state_Idle : Outport  Port=2  InitialOutput=[0]
  wires:
  Auto_Idle/1(cmd_AutoIdle) -> cmd_AutoIdle/1
  state_Engine/1 -> Auto_Idle/1(state_Engine)
  state_Console/1 -> Auto_Idle/2(state_Console)
  psi_LoadSense/1 -> Auto_Idle/3(psi_LoadSense)
  state_AutoIdleEnable/1 -> Auto_Idle/4(state_AutoIdleEnable)
  Constant1/1 -> CompareToConstant1/1
  CompareToConstant1/1 -> Switch/2
  Constant2/1 -> Switch/1
  Switch/1 -> Auto_Idle/5(percent_Throttle)
  percent_Throttle/1 -> Switch/3
  Auto_Idle/2(state_Idle) -> state_Idle/1
  AutoIdleTime/1 -> Auto_Idle/6(AutoIdleTime)

== system MX_Gateway/MX_Gtwy_Control/System/CAN_Throttle/CAN_Throttle ==
  note: Medium and Large Frame have throttle extended at low idle
      | Small Frame has throttle retracted at low idle
  fdbk_CANThrottle : Inport
  flag_InitEERead : Inport  Port=2
  pos_Throttle : Inport  Port=3
  state_Engine : Inport  Port=4
  state_CommThrottle : Inport  Port=5
  cmd_Throttle : Inport  Port=6
  Enable : EnablePort  StatesWhenEnabling=reset
  Abs : Abs  OutDataTypeStr=uint16
  CAN_Throttle_Actuator : Stateflow chart  TreatAsAtomicUnit=on
  CompareToConstant1 : simulink/Logic and Bit Operations/Compare To Constant  relop===  const=NON_FUNCTIONAL  OutDataTypeStr=boolean  ZeroCross=off
  Data Store Read : DataStoreRead  DataStoreName=ee_ThrottleCalRetracted  SampleTime=srate100
  Data Store Read1 : DataStoreRead  DataStoreName=ee_ThrottleCalExtended  SampleTime=srate100
  Mux : Mux  Inputs=2
  Mux1 : Mux  Inputs=2
  Sum : Sum  Inputs=|+-  OutDataTypeStr=int16
  Switch : Switch  Criteria=u2 ~= 0
  Switch1 : Switch  Criteria=u2 ~= 0
  THROTTLE_HIGH_IDLE : Constant  Value=1000  OutDataTypeStr=uint16  SampleTime=srate100
  THROTTLE_LOW_IDLE : Constant  Value=0  OutDataTypeStr=uint16  SampleTime=srate100
  THROTTLE_RETRACTED1 : Constant  Value=CD_CAN_THROTTLE_REVERSE  OutDataTypeStr=uint8  SampleTime=srate100
  Throttle_Cal_Scaling : simulink/Lookup Tables/Lookup Table Dynamic  LookUpMeth=Interpolation-Use End Values  OutDataType=fixdt('uint16')  OutScaling=[]  OutputDataTypeScalingMode=Specify via dialog  OutDataTypeStr=fixdt('uint16')  DoSatur=off
      settings: SourceProductName=Simulink
  cmd_CANThrottle : Outport  OutputWhenDisabled=reset  InitialOutput=0
  state_CANThrottle : Outport  Port=2  OutputWhenDisabled=reset  InitialOutput=INIT_NORMAL
  state_CANThrottleCalDiag : Outport  Port=3  OutputWhenDisabled=reset  InitialOutput=0
  wires:
  fdbk_CANThrottle/1 -> Sum/2, CAN_Throttle_Actuator/2(fdbk_CANThrottle)
  CAN_Throttle_Actuator/1(cmd_CANThrottle) -> cmd_CANThrottle/1
  CAN_Throttle_Actuator/2(state_CANThrottle) -> state_CANThrottle/1
  CAN_Throttle_Actuator/3(state_CANThrottleCalDiag) -> state_CANThrottleCalDiag/1
  flag_InitEERead/1 -> CAN_Throttle_Actuator/3(flag_InitEERead)
  state_Engine/1 -> CAN_Throttle_Actuator/4(state_Engine)
  state_CommThrottle/1 -> CAN_Throttle_Actuator/5(state_CommThrottle)
  Sum/1 -> Abs/1
  Abs/1 -> CAN_Throttle_Actuator/1(pos_Error)
  pos_Throttle/1 -> CAN_Throttle_Actuator/6(pos_Throttle), Throttle_Cal_Scaling/1
  THROTTLE_LOW_IDLE/1 -> Mux/1
  THROTTLE_HIGH_IDLE/1 -> Mux/2
  Mux/1 -> Throttle_Cal_Scaling/2
  Mux1/1 -> Throttle_Cal_Scaling/3
  Throttle_Cal_Scaling/1 -> CAN_Throttle_Actuator/7(scaled_ThrottlePos)
  cmd_Throttle/1 -> Sum/1
  THROTTLE_RETRACTED1/1 -> CompareToConstant1/1
  CompareToConstant1/1 -> Switch/2, Switch1/2
  Data Store Read1/1 -> Switch/1, Switch1/3
  Switch/1 -> Mux1/1
  Data Store Read/1 -> Switch1/1, Switch/3
  Switch1/1 -> Mux1/2

== system MX_Gateway/MX_Gtwy_Control/System/EMEA Control Mode/ModeDisplay ==
  note: Stuff to add blinking display at startup reminding operator of aux mode last time machine was on
  dout_GlowPlugs : Inport
  state_AuxEnable : Inport  Port=2
  flag_InitEERead : Inport  Port=3
  state_System : Inport  Port=4
  state_Console : Inport  Port=5
  state_Engine : Inport  Port=6
  mode_LeftControl : Inport  Port=7
  mode_RightControl : Inport  Port=8
  DisplayAuxMode : Stateflow chart  TreatAsAtomicUnit=on
  disp_LeftControl : Outport
  disp_RightControl : Outport  Port=2
  flag_AuxEnableFlash : Outport  Port=3
  wires:
  mode_LeftControl/1 -> DisplayAuxMode/1(mode_LeftControl)
  mode_RightControl/1 -> DisplayAuxMode/2(mode_RightControl)
  state_AuxEnable/1 -> DisplayAuxMode/3(state_AuxEnable)
  DisplayAuxMode/1(disp_LeftControl) -> disp_LeftControl/1
  DisplayAuxMode/2(disp_RightControl) -> disp_RightControl/1
  DisplayAuxMode/3(flag_AuxEnableFlash) -> flag_AuxEnableFlash/1
  flag_InitEERead/1 -> DisplayAuxMode/4(flag_InitEERead)
  dout_GlowPlugs/1 -> DisplayAuxMode/5(dout_GlowPlugs)
  state_Console/1 -> DisplayAuxMode/6(state_Console)
  state_Engine/1 -> DisplayAuxMode/7(state_Engine)
  state_System/1 -> DisplayAuxMode/8(state_System)

== system MX_Gateway/MX_Gtwy_Control/System/EMEA Control Mode/left_valid ==
  ratio : Inport
  state : Inport  Port=2
  Compare To Constant3 : simulink/Logic and Bit Operations/Compare To Constant  relop=>=  const=CD_EMEA_POT_CAL_DEADBAND_LOW  OutDataTypeStr=boolean
  Compare To Constant4 : simulink/Logic and Bit Operations/Compare To Constant  relop=<=  const=CD_EMEA_POT_CAL_DEADBAND_HIGH  OutDataTypeStr=boolean
  Compare To Constant5 : simulink/Logic and Bit Operations/Compare To Constant  relop=~=  const=OORH  OutDataTypeStr=boolean
  Compare To Constant6 : simulink/Logic and Bit Operations/Compare To Constant  relop=~=  const=OORL  OutDataTypeStr=boolean
  Logical Operator2 : Logic  Inputs=4  AllPortsSameDT=off  OutDataTypeStr=boolean
  valid : Outport
  wires:
  state/1 -> Compare To Constant6/1, Compare To Constant5/1
  ratio/1 -> Compare To Constant4/1, Compare To Constant3/1
  Logical Operator2/1 -> valid/1
  Compare To Constant6/1 -> Logical Operator2/4
  Compare To Constant3/1 -> Logical Operator2/1
  Compare To Constant5/1 -> Logical Operator2/3
  Compare To Constant4/1 -> Logical Operator2/2

== system MX_Gateway/MX_Gtwy_Control/System/EMEA Control Mode/right_valid ==
  ratio : Inport
  state : Inport  Port=2
  Compare To Constant3 : simulink/Logic and Bit Operations/Compare To Constant  relop=>=  const=CD_EMEA_POT_CAL_DEADBAND_LOW  OutDataTypeStr=boolean
  Compare To Constant4 : simulink/Logic and Bit Operations/Compare To Constant  relop=<=  const=CD_EMEA_POT_CAL_DEADBAND_HIGH  OutDataTypeStr=boolean
  Compare To Constant5 : simulink/Logic and Bit Operations/Compare To Constant  relop=~=  const=OORH  OutDataTypeStr=boolean
  Compare To Constant6 : simulink/Logic and Bit Operations/Compare To Constant  relop=~=  const=OORL  OutDataTypeStr=boolean
  Logical Operator2 : Logic  Inputs=4  AllPortsSameDT=off  OutDataTypeStr=boolean
  valid : Outport
  wires:
  state/1 -> Compare To Constant6/1, Compare To Constant5/1
  ratio/1 -> Compare To Constant4/1, Compare To Constant3/1
  Logical Operator2/1 -> valid/1
  Compare To Constant6/1 -> Logical Operator2/4
  Compare To Constant3/1 -> Logical Operator2/1
  Compare To Constant5/1 -> Logical Operator2/3
  Compare To Constant4/1 -> Logical Operator2/2

== system MX_Gateway/MX_Gtwy_Control/Inputs/Aux_Pot/Aux_Pot/Pot Ratio ==
  ain_AuxPot : Inport
  ain_SensorSupply2 : Inport  Port=2
  Gain : Gain  Gain=CD_AUXPOT_RATIO_GAIN  ParamDataTypeStr=uint(16)  OutDataTypeStr=uint(16)
  Product : Product  Inputs=*/  OutDataTypeStr=fixdt(0,16,15)  RndMeth=Simplest  LockScale=on
  rm_AuxPot : Outport
  wires:
  Product/1 -> Gain/1
  ain_AuxPot/1 -> Product/1
  Gain/1 -> rm_AuxPot/1
  ain_SensorSupply2/1 -> Product/2

== system MX_Gateway/MX_Gtwy_Control/Inputs/EMEA_Left_Control/Normal_Pot/Pot Ratio ==
  ain_LeftPot : Inport
  ain_SensorSupply2 : Inport  Port=2
  CompareToConstant2 : simulink/Logic and Bit Operations/Compare To Constant  relop===  const=NON_FUNCTIONAL  OutDataTypeStr=boolean  ZeroCross=off
  Constant1 : Constant  Value=CD_EMEA_CONTROLS_REVERSE
  Constant2 : Constant  Value=1000  OutDataTypeStr=uint16
  Gain : Gain  Gain=CD_OFFSET_POT_RATIO_GAIN  ParamDataTypeStr=uint(16)  OutDataTypeStr=uint(16)
  Product : Product  Inputs=*/  OutDataTypeStr=fixdt(0,16,15)  RndMeth=Simplest  LockScale=on
  Saturation : Saturate  UpperLimit=1000  LowerLimit=0
  Sum : Sum  Inputs=-+|
  Switch : Switch  Criteria=u2 ~= 0
  rm_LeftPot : Outport
  raw_LeftPot : Outport  Port=2
  wires:
  Product/1 -> Gain/1
  ain_LeftPot/1 -> Product/1
  ain_SensorSupply2/1 -> Product/2
  Constant1/1 -> CompareToConstant2/1
  Sum/1 -> Switch/3
  CompareToConstant2/1 -> Switch/2
  Constant2/1 -> Sum/2
  Gain/1 -> raw_LeftPot/1, Saturation/1
  Saturation/1 -> Sum/1, Switch/1
  Switch/1 -> rm_LeftPot/1

== system MX_Gateway/MX_Gtwy_Control/Inputs/EMEA_Right_Control/Right Pot/Pot Ratio ==
  ain_RightPot : Inport
  ain_SensorSupply2 : Inport  Port=2
  CompareToConstant2 : simulink/Logic and Bit Operations/Compare To Constant  relop===  const=NON_FUNCTIONAL  OutDataTypeStr=boolean  ZeroCross=off
  Constant1 : Constant  Value=CD_EMEA_CONTROLS_REVERSE
  Constant2 : Constant  Value=1000  OutDataTypeStr=uint16
  Gain : Gain  Gain=CD_AUXPOT_RATIO_GAIN  ParamDataTypeStr=uint(16)  OutDataTypeStr=uint(16)
  Product : Product  Inputs=*/  OutDataTypeStr=fixdt(0,16,15)  RndMeth=Simplest  LockScale=on
  Saturation : Saturate  UpperLimit=1000  LowerLimit=0
  Sum : Sum  Inputs=-+|
  Switch : Switch  Criteria=u2 ~= 0
  raw_RightPot : Outport
  rm_RightPot : Outport  Port=2
  wires:
  Product/1 -> Gain/1
  ain_RightPot/1 -> Product/1
  ain_SensorSupply2/1 -> Product/2
  Constant1/1 -> CompareToConstant2/1
  Sum/1 -> Switch/3
  Switch/1 -> rm_RightPot/1
  CompareToConstant2/1 -> Switch/2
  Constant2/1 -> Sum/2
  Gain/1 -> raw_RightPot/1, Saturation/1
  Saturation/1 -> Sum/1, Switch/1
